simulink model "HDVSim_XNG_Hybrid_Truck"  (R2019b) (continued)
== system HDVSim_XNG_Hybrid_Truck/Driving cycle == (continued)
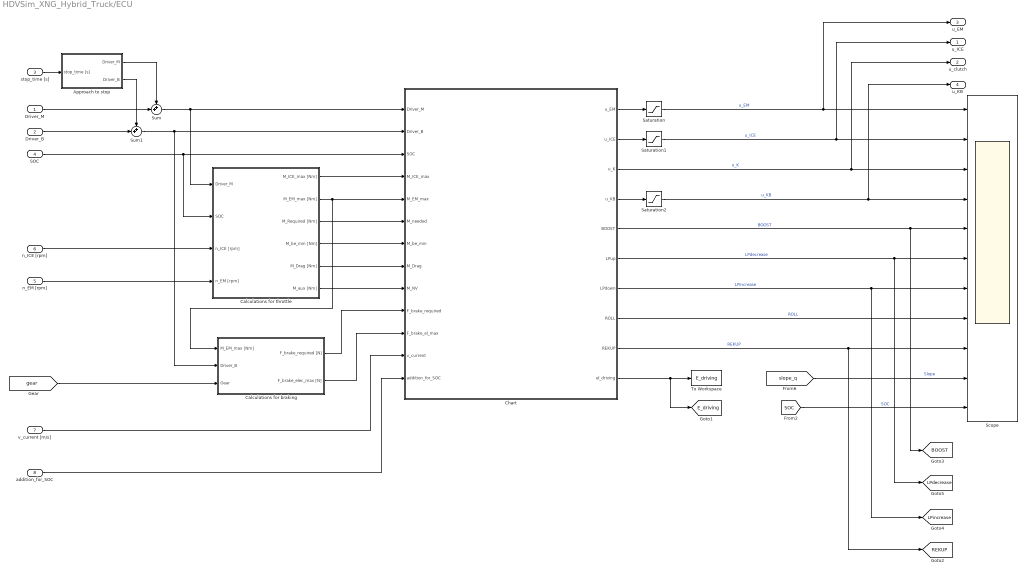
== system HDVSim_XNG_Hybrid_Truck/ECU ==
  Driver_M : Inport
  Driver_B : Inport  Port=2
  stop_time [s] : Inport  Port=3
  SOC : Inport  Port=4
  n_EM [rpm] : Inport  Port=5
  n_ICE [rpm] : Inport  Port=6
  v_current [m/s] : Inport  Port=7
  addition_for_SOC : Inport  Port=8
  Approach to stop : Subsystem
  Calculations for braking : Subsystem
  Calculations for throttle : Subsystem
  Chart : Stateflow chart  TreatAsAtomicUnit=on
  Saturation : Saturate  UpperLimit=1  LowerLimit=-1
  Saturation1 : Saturate  UpperLimit=1  LowerLimit=0
  Saturation2 : Saturate  UpperLimit=1  LowerLimit=0
  Scope : Scope  NumInputPorts=11
  Sum : Sum  Inputs=++|
  Sum1 : Sum  Inputs=++|
  To Workspace : ToWorkspace  VariableName=E_driving  SampleTime=-1
      settings: Decimation=10
  u_ICE : Outport  VectorParamsAs1DForOutWhenUnconnected=off
  u_clutch : Outport  Port=2  VectorParamsAs1DForOutWhenUnconnected=off
  u_EM : Outport  Port=3  VectorParamsAs1DForOutWhenUnconnected=off
  u_KB : Outport  Port=4  VectorParamsAs1DForOutWhenUnconnected=off
  tag scope: BOOST=global, E_driving=global, LPdecrease=global, LPincrease=global, REKUP=global
  wires:
  Chart/1(u_EM) -> Saturation/1
  Chart/2(u_ICE) -> Saturation1/1
  Chart/4(u_KB) -> Saturation2/1
  Sum1/1 -> Chart/2(Driver_B), Calculations for braking/2(Driver_B)
  Sum/1 -> Chart/1(Driver_M), Calculations for throttle/1(Driver_M)
  Chart/3(u_K) -> u_clutch/1, Scope/3  "u_K"
  Calculations for braking/2(F_brake_elec_max [N]) -> Chart/11(F_brake_el_max)
  Calculations for braking/1(F_brake_required [N]) -> Chart/10(F_brake_required)
  SOC/1 -> Chart/3(SOC), Calculations for throttle/2(SOC)
  n_ICE [rpm]/1 -> Calculations for throttle/3(n_ICE [rpm])
  Calculations for throttle/1(M_ICE_max [Nm]) -> Chart/4(M_ICE_max)
  Calculations for throttle/3(M_Required [Nm]) -> Chart/6(M_needed)
  Saturation1/1 -> u_ICE/1, Scope/2  "u_ICE"
  Saturation2/1 -> u_KB/1, Scope/4  "u_KB"
  Calculations for throttle/5(M_Drag [Nm]) -> Chart/8(M_Drag)
  Calculations for throttle/4(M_be_min [Nm]) -> Chart/7(M_be_min)
  Chart/5(BOOST) -> Scope/5, [BOOST]  "BOOST"
  Chart/8(ROLL) -> Scope/8  "ROLL"
  Chart/9(REKUP) -> [REKUP], Scope/9  "REKUP"
  Chart/7(LPdown) -> [LPincrease], Scope/7  "LPincrease"
  [slope_q] -> Scope/10  "Slope"
  Calculations for throttle/6(M_aux [Nm]) -> Chart/9(M_NV)
  Chart/6(LPup) -> [LPdecrease], Scope/6  "LPdecrease"
  Calculations for throttle/2(M_EM_max [Nm]) -> Chart/5(M_EM_max), Calculations for braking/1(M_EM_max [Nm])
  [SOC] -> Scope/11  "SOC"
  Saturation/1 -> u_EM/1, Scope/1  "u_EM"
  stop_time [s]/1 -> Approach to stop/1(stop_time [s])
  Approach to stop/2(Driver_B) -> Sum1/1
  Approach to stop/1(Driver_M) -> Sum/1
  Driver_M/1 -> Sum/2
  Driver_B/1 -> Sum1/2
  n_EM [rpm]/1 -> Calculations for throttle/4(n_EM [rpm])
  [gear] -> Calculations for braking/3(Gear)
  v_current [m/s]/1 -> Chart/12(v_current)
  Chart/10(el_driving) -> [E_driving], To Workspace/1
  addition_for_SOC/1 -> Chart/13(addition_for_SOC)

== system HDVSim_XNG_Hybrid_Truck/Electric motor ==
  note: Electric torque
  note: Effective motor power
  u_EM : Inport
  n_EM [rpm] : Inport  Port=2
  U_Bat [V] : Inport  Port=3
  Abs : Abs
  Abs1 : Abs
  Divide : Product  Inputs=*/
  Electric machine efficiency mapping : Lookup_n-D  BreakpointsForDimension1=em.efficiency.torque  BreakpointsForDimension2=em. efficiency.speed  Table=em.efficiency.characteristic_map  RndMeth=Simplest  InputSameDT=on
  Electric machine efficiency mapping2 : Lookup_n-D  BreakpointsForDimension1=em.efficiency.torque  BreakpointsForDimension2=em. efficiency.speed  Table=em.efficiency.characteristic_map  RndMeth=Simplest  InputSameDT=on
  Gain : Gain  Gain=2*pi/60
  Machine current demand : Subsystem
  Machine torque demand : Subsystem
  Math Function : Math  Operator=reciprocal  SaturateOnIntegerOverflow=on
  Product1 : Product
  Product2 : Product  Inputs=3
  Saturation : Saturate  UpperLimit=Bat.Useable_capacity / Bat.Useable_range*10  LowerLimit=-Bat.Useable_capacity / Bat.Useable_range*10
  Saturation1 : Saturate  UpperLimit=inf  LowerLimit=1
  Speed-torrque characteristics : Lookup_n-D  NumberOfTableDimensions=1  BreakpointsForDimension1=em.speed  Table=em.trq  ExtrapMethod=Clip  RndMeth=Simplest  InputSameDT=on
  Switch : Switch
  M_EM [Nm] : Outport  VectorParamsAs1DForOutWhenUnconnected=off
  I_EM_current [A] : Outport  Port=2  VectorParamsAs1DForOutWhenUnconnected=off
  tag scope: M_EM=global, n_EM=global, u_EM=global
  wires:
  Speed-torrque characteristics/1 -> Product1/1  "M_EM_max"
  u_EM/1 -> Product1/2, [u_EM]
  U_Bat [V]/1 -> Divide/2, Machine torque demand/4(U_Bat [V]), Machine current demand/4(U_Bat [V])
  Machine current demand/1(I_target [A]) -> Saturation/1
  Saturation/1 -> Machine torque demand/2(I_current [A])
  Machine torque demand/1(M_EM [Nm]) -> [M_EM], M_EM [Nm]/1, Switch/2, Abs/1, Product2/3
  Gain/1 -> Product2/2
  Divide/1 -> I_EM_current [A]/1
  Math Function/1 -> Switch/1
  Electric machine efficiency mapping2/1 -> Switch/3, Math Function/1  "Eta_EM"
  Switch/1 -> Product2/1
  Product2/1 -> Divide/1
  Abs/1 -> Electric machine efficiency mapping2/1
  Product1/1 -> Machine current demand/2(M_EM_target [Nm]), Abs1/1  "M_EM_target"
  n_EM [rpm]/1 -> Electric machine efficiency mapping/2, Saturation1/1, Electric machine efficiency mapping2/2, Machine torque demand/1(n_EM [rpm]), Machine current demand/1("n_EM "), [n_EM], Speed-torrque characteristics/1
  Abs1/1 -> Electric machine efficiency mapping/1
  Electric machine efficiency mapping/1 -> Machine current demand/3(Eff_EM), Machine torque demand/3(Eff_EM)  "ETA_EM"
  Saturation1/1 -> Gain/1

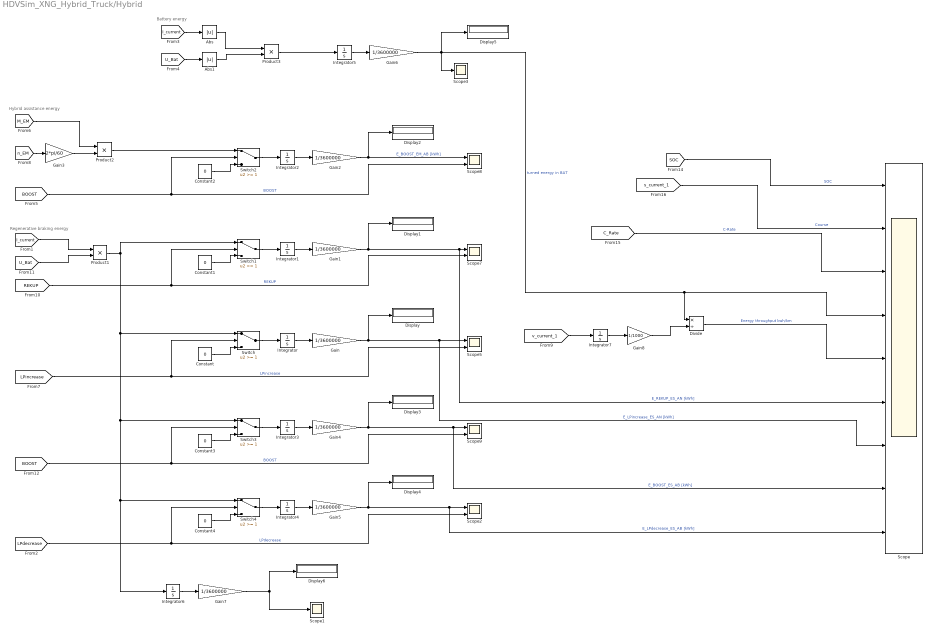
== system HDVSim_XNG_Hybrid_Truck/Hybrid ==
  note: Battery energy
  note: Hybrid assistance energy
  note: Regenerative braking energy
  Abs : Abs
  Abs1 : Abs
  Constant{,1..4} (5 alike) : Constant  Value=0
  Display{,1..6} (7 alike) : Display
  Divide : Product  Inputs=*/
  Gain{,1..2,4..7} (7 alike) : Gain  Gain=1/3600000
  Gain3 : Gain  Gain=2*pi/60
  Gain8 : Gain  Gain=1/1000
  Integrator{,1..6} (7 alike) : Integrator
  Integrator7 : Integrator  InitialCondition=0.00000001
  Product1 : Product
  Product2 : Product
  Product3 : Product
  Scope : Scope  NumInputPorts=9
  Scope1 : Scope
  Scope{2,5,7..9} (5 alike) : Scope  NumInputPorts=2
  Scope3 : Scope
  Switch{,1..4} (5 alike) : Switch  Threshold=1
  wires:
  Product1/1 -> Switch1/1, Switch/1, Switch3/1, Integrator6/1, Switch4/1
  Gain5/1 -> Scope/9, Scope2/1, Display4/1  "E_LPdecrease_ES_AB [kWh]"
  Constant4/1 -> Switch4/3
  Integrator4/1 -> Gain5/1
  Switch4/1 -> Integrator4/1
  [LPdecrease] -> Scope2/2, Switch4/2  "LPdecrease"
  Gain4/1 -> Scope/8, Scope9/1, Display3/1  "E_BOOST_ES_AB [kWh]"
  Constant3/1 -> Switch3/3
  Integrator3/1 -> Gain4/1
  Switch3/1 -> Integrator3/1
  [BOOST] -> Scope9/2, Switch3/2  "BOOST"
  Gain3/1 -> Product2/2
  [n_EM] -> Gain3/1
  Gain2/1 -> Scope8/1, Display2/1  "E_BOOST_EM_AB [kWh]"
  Constant2/1 -> Switch2/3
  Integrator2/1 -> Gain2/1
  Switch2/1 -> Integrator2/1
  [BOOST] -> Scope8/2, Switch2/2  "BOOST"
  Product2/1 -> Switch2/1
  Gain1/1 -> Scope/6, Scope7/1, Display1/1  "E_REKUP_ES_AN [kWh]"
  Constant1/1 -> Switch1/3
  Integrator1/1 -> Gain1/1
  Switch1/1 -> Integrator1/1
  [REKUP] -> Scope7/2, Switch1/2  "REKUP"
  [U_Bat] -> Product1/2
  [I_current] -> Product1/1
  Gain/1 -> Scope/7, Scope5/1, Display/1  "E_LPincrease_ES_AN [kWh]"
  Constant/1 -> Switch/3
  Integrator/1 -> Gain/1
  Switch/1 -> Integrator/1
  [LPincrease] -> Scope5/2, Switch/2  "LPincrease"
  [M_EM] -> Product2/1
  Gain7/1 -> Display6/1, Scope1/1
  Integrator6/1 -> Gain7/1
  [U_Bat] -> Abs1/1
  [I_current] -> Abs/1
  Product3/1 -> Integrator5/1
  Integrator5/1 -> Gain6/1
  Abs/1 -> Product3/1
  Abs1/1 -> Product3/2
  [SOC] -> Scope/1  "SOC"
  [s_current_1] -> Scope/2  "Course"
  [C_Rate] -> Scope/3  "C-Rate"
  Divide/1 -> Scope/5  "Energy throughput kwh/km"
  Gain6/1 -> Scope3/1, Display5/1, Divide/1, Scope/4  "turned energy in BAT"
  [v_current_1] -> Integrator7/1
  Integrator7/1 -> Gain8/1
  Gain8/1 -> Divide/2

== system HDVSim_XNG_Hybrid_Truck/ICE engine ==
  note: Engine loading
  note: Consumption calculations
  u_ICE : Inport
  n_ICE [rpm] : Inport  Port=2
  Additional consumptions : Subsystem
  Consumption : Subsystem
  Drag torque : Lookup  InputValues=engine.drag_torque.speed  Table=engine.drag_torque.trq  LookUpMeth=Interpolation-Use End Values
  Full load curve : Lookup  InputValues=engine.full_load.speed  Table=engine.full_load.trq  LookUpMeth=Interpolation-Use End Values
  MinMax : MinMax  Inputs=2
  Product1 : Product
  Sum : Sum  Inputs=|+-
  Sum1 : Sum  Inputs=|++
  maximum engine torque due to tire slip : Subsystem
  M_ICE [Nm] : Outport  VectorParamsAs1DForOutWhenUnconnected=off
  tag scope: M_mot=global, n_mot=global
  wires:
  n_ICE [rpm]/1 -> Full load curve/1, Consumption/2(n [rpm]), Additional consumptions/2(n_clutch_en [rpm]), [n_mot], Drag torque/1
  Sum/1 -> Product1/2
  Product1/1 -> Sum1/1
  Drag torque/1 -> Sum1/2, Sum/2  "M_Drag"
  Additional consumptions/1(M_ICE_aftr_aux [Nm]) -> M_ICE [Nm]/1
  u_ICE/1 -> Product1/1
  Full load curve/1 -> MinMax/2  "M_VM_max"
  Sum1/1 -> Consumption/1(M [Nm]), [M_mot], Additional consumptions/1(M_ICE_bfor_aux [Nm])
  maximum engine torque due to tire slip/1(M_mot_max_traction [Nm]) -> MinMax/1
  MinMax/1 -> Sum/1

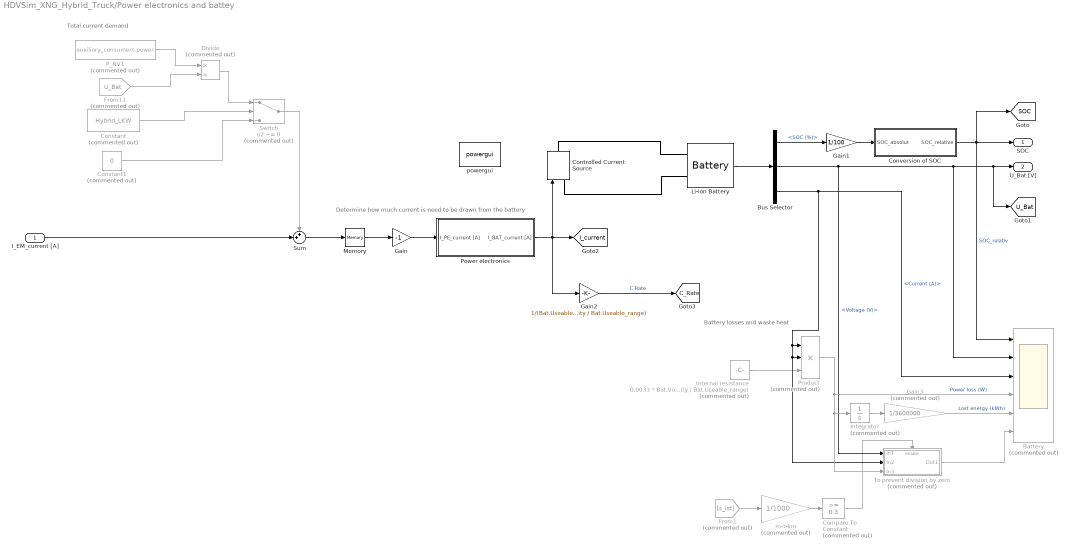
== system HDVSim_XNG_Hybrid_Truck/Power electronics and battey ==
  note: Determine how much current is need to be drawn from the battery
  note: Battery losses and waste heat
  note: Total current demand
  I_EM_current [A] : Inport
  Battery : Scope  Commented=on  NumInputPorts=6
  Bus Selector : BusSelector  OutputSignals=SOC (%),Voltage (V),Current (A)
  Compare To Constant : simulink/Logic and Bit Operations/Compare To Constant  Commented=on  relop=>=  const=0.3  OutDataTypeStr=uint8  ZeroCross=off
  Constant : Constant  Commented=on  Value=Hybrid_LKW
  Constant1 : Constant  Commented=on  Value=0
  Controlled Current Source : powerlib/Electrical Sources/Controlled Current Source  Initialize=off  Source_Type=DC  Amplitude=0  Phase=0  Frequency=0  Measurements=None
      settings: SourceProductName=Simscape Electrical
  Conversion of SOC : Subsystem
  Divide : Product  Commented=on  Inputs=*/
  From1 : From  Commented=on  GotoTag=s_ist  TagVisibility=global
      settings: CloseFcn=tagdialog Close
  From11 : From  Commented=on  GotoTag=U_Bat  TagVisibility=global
  Gain : Gain  Gain=-1
  Gain1 : Gain  Gain=1/100
  Gain2 : Gain  Gain=1/(Bat.Useable_capacity / Bat.Useable_range)
  Gain3 : Gain  Commented=on  Gain=1/3600000
  Integrator : Integrator  Commented=on
  Internal resistance : Constant  Commented=on  Value=0.0033 * Bat.Voltage / (Bat.Useable_capacity / Bat.Useable_range)
  Li-ion Battery : electricdrivelib/Extra Sources/Battery  BatType=Lithium-Ion  ShowTempParam=off  ThermalPreset=no  ShowAgeParam=off  NomV=Bat.Voltage  NomQ=Bat.Useable_capacity / Bat.Useable_range  SOC=(Bat.SOC_start *Bat.Useable_range+(0.5-Bat.Useable_range/2))* 100  Batt_Tr=30  PresetModel=off  MaxQ=Bat.Useable_capacity / Bat.Useable_range *8077/7500  MinV=487.5  FullV=Bat.Voltage * 1.178  Dis_rate=Bat.Useable_capacity / Bat.Useable_range * 1  R=0.0033 * Bat.Voltage / (Bat.Useable_capacity / Bat.Useable_range)  Normal_OP=Bat.Useable_capacity / Bat.Useable_range * 0.962  expZone="[Bat.Voltage*4339/4000     Bat.Useable_capacity / Bat.Useable_range/5]"  current=[5 25 50 250]  Units=Ampere-hour  Tci=20  NomT=20  T2=-30  MaxQ2=4.8  FullV2=7.1  Normal_OP2=5.72  expZone2="[6.58  1]"  Rca=0.6  ti=1000  Dp=0  Neq0=0  Tsim=1e6  Ta1=25  Qeol=5.4*0.9  Reol=0.013333*1.2  Char_age=[2.3478, 3]  Dis_age=[2.3478, 10]  N1=1500  N2=10500  N3=1000  N4=1400  Ta2=45  N5=950
      settings: SourceProductName=Simscape Electrical
  Memory : Memory
  P_NV1 : Constant  Commented=on  Value=auxiliary_consumers.power
  Power electronics : Subsystem
  Product : Product  Commented=on  Inputs=3
  Sum : Sum  Inputs=++|
  Switch : Switch  Commented=on  Criteria=u2 ~= 0
  To prevent division by zero : Subsystem  Commented=on
  m->km : Gain  Commented=on  Gain=1/1000
  powergui : powerlib/powergui  Priority=1  SimulationMode=Discrete  SampleTime=sample_time  frequency=60  Iterations=50  frequencyindice=0  Pbase=100e6  ErrMax=1e-4  UnitsV=kV  UnitsW=MW  buscounter=0  FunctionMessages=off  echomessages=off  EnableUseOfTLC=off  CurrentSourceSwitches=off  DisableSnubberDevices=off  DisableRonSwitches=off  DisableVfSwitches=off  DisplayEquations=off  AutomaticDiscreteSolvers=off  SolverType=Tustin  Interpol=off  ExternalGateDelay=off  methode=off  Ts=100  x0status=blocks  SPID=off  SwTol=0  HookPort=off  NonlinearTolerance=1e-4  nMaxIteration=100  ContinueOnMaxIteration=off  RestoreLinks=warning  ResistiveCurrentMeasurement=off  Frange=[0:2:500]  Ylog=off  Xlog=on  ShowGrid=off  save=off  variable=ZData  structure=0  ZoomFFT=off  StartTime=0.0  cycles=1  DisplayStyle=1  fundamental=60  FreqAxis=off  MaxFrequency=1000  frequencyindicesteady=1  RmsSteady=1  display=off
      settings: SourceProductName=Simscape Electrical
  SOC : Outport  VectorParamsAs1DForOutWhenUnconnected=off
  U_Bat [V] : Outport  Port=2  VectorParamsAs1DForOutWhenUnconnected=off
  tag scope: C_Rate=global, I_current=global, SOC=global, U_Bat=global
  wires:
  Li-ion Battery/1 -> Bus Selector/1
  Bus Selector/1 -> Gain1/1  "<SOC (%)>"
  Gain1/1 -> Conversion of SOC/1(SOC_absolut)
  Memory/1 -> Gain/1
  Gain/1 -> Power electronics/1(I_PE_current [A])
  Power electronics/1(I_BAT_current [A]) -> Gain2/1, Controlled Current Source/1, [I_current]
  Gain2/1 -> [C_Rate]  "C Rate"
  Gain3/1 -> Battery/5  "Lost energy (kWh)"
  Compare To Constant/1 -> To prevent division by zero/Enable
  To prevent division by zero/1(Out1) -> Battery/6
  Integrator/1 -> Gain3/1
  From1/1 -> m->km/1
  Internal resistance/1 -> Product/3
  Bus Selector/3 -> Battery/3, To prevent division by zero/2(In2), Product/2, Product/1  "<Current (A)>"
  Bus Selector/2 -> To prevent division by zero/1(In1), Battery/2, [U_Bat], U_Bat [V]/1  "<Voltage (V)>"
  Product/1 -> Battery/4, To prevent division by zero/3(In3), Integrator/1  "Power loss (W)"
  m->km/1 -> Compare To Constant/1
  Conversion of SOC/1(SOC_relative) -> Battery/1, [SOC], SOC/1  "SOC_relativ"
  From11/1 -> Divide/2
  P_NV1/1 -> Divide/1
  Divide/1 -> Switch/1
  Constant1/1 -> Switch/3
  Switch/1 -> Sum/1
  Constant/1 -> Switch/2
  Sum/1 -> Memory/1
  I_EM_current [A]/1 -> Sum/2
  connections:
  Li-ion Battery/LConn1 -- Controlled Current Source/RConn1
  Li-ion Battery/LConn2 -- Controlled Current Source/LConn1

== system HDVSim_XNG_Hybrid_Truck/Tires ==
  M_tire [Nm] : Inport
  v_current [m/s] : Inport  Port=2
  F=M/r : Product  Inputs=*/
  Tire radius : Constant  Value=tires.radius
  "phi. -> n[1/min]" : Gain  Gain=60/2/pi
  phi. = v/r : Product  Inputs=/*
  n_tire [rpm] : Outport  VectorParamsAs1DForOutWhenUnconnected=off
  F_x [N] : Outport  Port=2  VectorParamsAs1DForOutWhenUnconnected=off
  wires:
  phi. = v/r/1 -> "phi. -> n[1/min]"/1
  "phi. -> n[1/min]"/1 -> n_tire [rpm]/1
  Tire radius/1 -> phi. = v/r/1, F=M/r/2
  v_current [m/s]/1 -> phi. = v/r/2
  M_tire [Nm]/1 -> F=M/r/1
  F=M/r/1 -> F_x [N]/1

== system HDVSim_XNG_Hybrid_Truck/Transmission ==
  note: Transmission torque multiplication through the different gears
  M_EM [Nm] : Inport
  M_clutch_dis [Nm] : Inport  Port=2
  n_Gearbox [rpm] : Inport  Port=3
  alpha : Inport  Port=4
  Determine when shifting occurs : Subsystem  TreatAsAtomicUnit=on
  Memory1 : Memory
  Number of gears : Constant  Value=length(transmission.ratios)
  Saturation : Saturate  UpperLimit=2000  LowerLimit=0
  Shifting strategy : Stateflow chart  TreatAsAtomicUnit=on
  Sum : Sum
  Torque flow through the transmission : Subsystem
  ZKU : Subsystem
  init_gear : Constant  Value=transmission.init_gear
  n_clutch_dis [rpm] : Outport  VectorParamsAs1DForOutWhenUnconnected=off
  M_Gearbox [Nm] : Outport  Port=2  VectorParamsAs1DForOutWhenUnconnected=off
  tag scope: gear=global, i_gearbox=global, recommend_DOWN_now=global, recommend_UP_now=global
  wires:
  n_Gearbox [rpm]/1 -> Torque flow through the transmission/2(n_gearbox_out [1/min]), Determine when shifting occurs/2(n_Getr_aus [1/min])
  Torque flow through the transmission/2(M_gearbox_out [Nm]) -> [M_gear_out], M_Gearbox [Nm]/1
  Torque flow through the transmission/1(n_gearbox_in [1/min]) -> Saturation/1
  init_gear/1 -> Shifting strategy/1(init_gear)
  Determine when shifting occurs/27(check_torque_reseve_2lower) -> Shifting strategy/29(check_torque_reserve_2lower)
  Determine when shifting occurs/26(check_torque_reseve_2higher) -> Shifting strategy/28(check_torque_reserve_2higher)
  Determine when shifting occurs/1(reccommend_down_2higher) -> Shifting strategy/3(recommend_down_2higher)
  Determine when shifting occurs/2(reccommend_down_1higher) -> Shifting strategy/4(recommend_down_1higher)
  Determine when shifting occurs/3(reccommend_down_now) -> Shifting strategy/5(recommend_down_now), [recommend_DOWN_now]
  Determine when shifting occurs/4(reccommend_down_1lower) -> Shifting strategy/6(recommend_down_1lower)
  Determine when shifting occurs/5(reccommend_down_2lower) -> Shifting strategy/7(recommend_down_2llower)
  Determine when shifting occurs/6(reccommend_up_2higher) -> Shifting strategy/8(recommend_up_2higher)
  Determine when shifting occurs/7(reccommend_up_1higher) -> Shifting strategy/9(recommend_up_1higher)
  Determine when shifting occurs/8(reccommend_up_now) -> Shifting strategy/10(recommend_up_now), [recommend_UP_now]
  Determine when shifting occurs/9(reccommend_up_1lower) -> Shifting strategy/11(recommend_up_1lower)
  Determine when shifting occurs/10(reccommend_up_2lower) -> Shifting strategy/12(recommend_up_2lower)
  Determine when shifting occurs/11(check_n_min_2higher) -> Shifting strategy/13(check_n_min_2higher)
  Determine when shifting occurs/12(check_n_min_1higher) -> Shifting strategy/14(check_n_min_1higher)
  Determine when shifting occurs/13(check_n_min_now) -> Shifting strategy/15(check_n_min_now)
  Determine when shifting occurs/14(check_n_min_1lower) -> Shifting strategy/16(check_n_min_1lower)
  Determine when shifting occurs/15(check_n_min_2lower) -> Shifting strategy/17(check_n_min_2lower)
  Determine when shifting occurs/16(check_n_max_2higher) -> Shifting strategy/18(check_n_max_2higher)
  Determine when shifting occurs/17(check_n_max_1higher) -> Shifting strategy/19(check_n_max_1higher)
  Determine when shifting occurs/18(check_n_max_now) -> Shifting strategy/20(check_n_max_now)
  Determine when shifting occurs/19(check_n_max_1lower) -> Shifting strategy/21(check_n_max_1lower)
  Determine when shifting occurs/20(check_n_max_2lower) -> Shifting strategy/22(check_n_max_2lower)
  Determine when shifting occurs/21(check_M_max_2higher) -> Shifting strategy/23(check_M_max_2higher)  "check_M_max_2higher"
  Determine when shifting occurs/22(check_M_max_1higher) -> Shifting strategy/24(check_M_max_1higher)  "check_M_max_1higher"
  Determine when shifting occurs/23(check_M_max_now) -> Shifting strategy/25(check_M_max_now)  "check_M_max_now"
  Determine when shifting occurs/24(check_M_max_1lower) -> Shifting strategy/26(check_M_max_1lower)  "check_M_max_1lower"
  Determine when shifting occurs/25(check_M_max_2lower) -> Shifting strategy/27(check_M_max_2lower)  "check_M_max_2lower"
  Memory1/1 -> ZKU/1(Gear), Torque flow through the transmission/3(Gear), [Gear], [gear]
  M_clutch_dis [Nm]/1 -> Sum/2
  Sum/1 -> Torque flow through the transmission/1(M_gearbox_in [Nm])
  Torque flow through the transmission/3(i_gearbox) -> [i_gearbox]
  Saturation/1 -> n_clutch_dis [rpm]/1
  M_EM [Nm]/1 -> Determine when shifting occurs/4(M_EM [Nm]), Sum/1
  Number of gears/1 -> Shifting strategy/2(z)
  alpha/1 -> Determine when shifting occurs/5(alpha)
  Shifting strategy/1(gear) -> [gear]
  [gear] -> Memory1/1
  [Gear] -> Determine when shifting occurs/1(Gear)
  [M_gear_out] -> Determine when shifting occurs/3(M_Getr_aus [Nm])

== system HDVSim_XNG_Hybrid_Truck/Vehicle ==
  note: Net tractive force applied on the vehicle
  note: Vehicle acceleration
  F_x [N] : Inport
  u_KB : Inport  Port=2
  alpha [rad] : Inport  Port=3
  Add : Sum  Inputs=-+-
  Breaking force [N] : Product  RndMeth=Zero
  Cargo weight : Constant  Value=vehicle.payload
  Driving resistances without acceleration : Subsystem
  F_brake_max : Constant  Value=vehicle.F_brake_max
  Integrator : Integrator
  Integrator1 : Integrator  InitialCondition=cycle.speed_init  LimitOutput=on  UpperSaturationLimit=33  LowerSaturationLimit=0
  Product2 : Product  Inputs=*/  RndMeth=Zero
  Product3 : Product  Inputs=**  RndMeth=Zero
  Saturation : Saturate  UpperLimit=15  LowerLimit=-15
  Sum1 : Sum  Inputs=|++
  Vehicle weight : Constant  Value=vehicle.mass
  v_current [m/s] : Outport  VectorParamsAs1DForOutWhenUnconnected=off
  s_current [m] : Outport  Port=2  VectorParamsAs1DForOutWhenUnconnected=off
  tag scope: s_current_1=global, v_current_1=global
  wires:
  alpha [rad]/1 -> Driving resistances without acceleration/1(alpha [rad])
  Add/1 -> Product2/1
  Driving resistances without acceleration/1(F_W [N]) -> Add/3
  F_x [N]/1 -> Add/2  "F_x"
  F_brake_max/1 -> Breaking force [N]/2
  Integrator/1 -> [s_current_1], s_current [m]/1  "s_ist"
  Cargo weight/1 -> Sum1/2
  Sum1/1 -> Product2/2
  Vehicle weight/1 -> Product3/2
  Product3/1 -> Sum1/1
  Integrator1/1 -> v_current [m/s]/1, Driving resistances without acceleration/2(v [m/s]), [v_current_1], Integrator/1  "v_ist"
  [lamda] -> Product3/1
  u_KB/1 -> Breaking force [N]/1
  Breaking force [N]/1 -> Add/1  "F_brake"
  Product2/1 -> Saturation/1  "a"
  Saturation/1 -> Integrator1/1

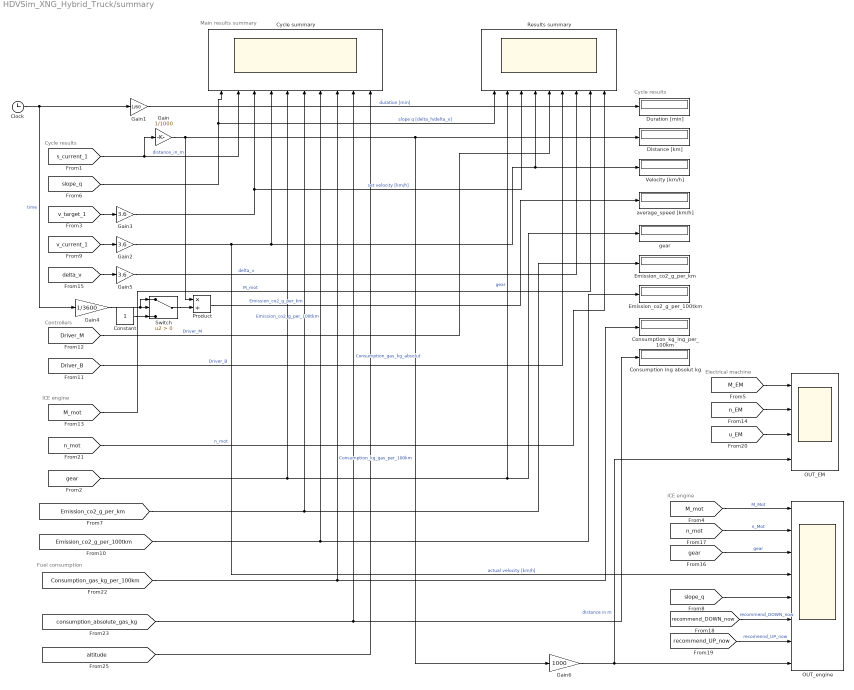
== system HDVSim_XNG_Hybrid_Truck/summary ==
  note: Main results summary
  note: Cycle results
  note: Controllers
  note: ICE engine
  note: Fuel consumption
  note: Cycle results
  note: Electrical machine
  note: ICE engine
  Clock : Clock
  Constant : Constant
  Consumption lng absolut kg : Display
  Consumption_kg_lng_per_100km : Display
  Cycle summary : Scope  NumInputPorts=10
  Distance [km] : Display
  Duration [min] : Display
  Emission_co2_g_per_100tkm : Display
  Emission_co2_g_per_km : Display
  Gain : Gain  Gain=1/1000
  Gain1 : Gain  Gain=1/60
  Gain2 : Gain  Gain=3.6
  Gain3 : Gain  Gain=3.6
  Gain4 : Gain  Gain=1/3600
  Gain5 : Gain  Gain=3.6
  Gain6 : Gain  Gain=1000
  OUT_EM : Scope  NumInputPorts=4
  OUT_engine : Scope  NumInputPorts=8
  Product : Product  Inputs=*/  RndMeth=Zero
  Results summary : Scope  NumInputPorts=9
  Switch : Switch  Criteria=u2 > Threshold
  Velocity [km/h] : Display
  average_speed [km/h] : Display
  gear : Display
  wires:
  [v_current_1] -> Gain2/1
  [s_current_1] -> Cycle summary/2, Gain/1  "distance_in_m"
  Gain/1 -> Gain6/1, Distance [km]/1, Product/1
  Clock/1 -> Gain1/1, Gain4/1  "time"
  Gain1/1 -> Duration [min]/1  "duration [min]"
  Gain2/1 -> Velocity [km/h]/1, Results summary/4, Cycle summary/4, OUT_engine/4  "actual velocity [km/h]"
  [gear] -> gear/1, Results summary/2, Cycle summary/5  "gear"
  [v_target_1] -> Gain3/1
  [slope_q] -> Results summary/1, Cycle summary/1  "slope q [delta_h/delta_x]"
  [Emission_co2_g_per_km] -> Emission_co2_g_per_km/1, Cycle summary/6  "Emission_co2_g_per_km"
  Gain3/1 -> Results summary/3, Cycle summary/3  "set velocity [km/h]"
  Gain4/1 -> Switch/1, Switch/2
  Product/1 -> average_speed [km/h]/1
  [Emission_co2_g_per_100tkm] -> Emission_co2_g_per_100tkm/1, Cycle summary/7  "Emission_co2_g_per_100tkm"
  [Driver_M] -> Results summary/5  "Driver_M"
  [Driver_B] -> Results summary/6  "Driver_B"
  [M_mot] -> Results summary/8  "M_mot"
  [delta_v] -> Gain5/1
  [M_mot] -> OUT_engine/1  "M_Mot"
  [n_mot] -> OUT_engine/2  "n_Mot"
  [gear] -> OUT_engine/3  "gear"
  [recommend_DOWN_now] -> OUT_engine/6  "recommend_DOWN_now"
  [recommend_UP_now] -> OUT_engine/7  "recomennd_UP_now"
  [slope_q] -> OUT_engine/5
  Gain5/1 -> Results summary/7  "delta_v"
  [n_mot] -> Results summary/9  "n_mot"
  [M_EM] -> OUT_EM/1
  [n_EM] -> OUT_EM/2
  [u_EM] -> OUT_EM/3
  [Consumption_gas_kg_per_100km] -> Consumption_kg_lng_per_100km/1, Cycle summary/8  "Consumption_kg_gas_per_100km"
  [consumption_absolute_gas_kg] -> Consumption lng absolut kg/1, Cycle summary/9  "Consumption_gas_kg_absolut"
  Switch/1 -> Product/2
  Constant/1 -> Switch/3
  [altitude] -> Cycle summary/10
  Gain6/1 -> OUT_EM/4, OUT_engine/8  "distance in m"

== system HDVSim_XNG_Hybrid_Truck/Active cruise control/Braking from upper speed limit ==
  v_target [m/s] : Inport
  Add3 : Sum  Inputs=+-
  Constant1 : Constant  Value=90/3.6
  Constant10 : Constant  Value=prospective_cruise_control
  Constant6 : Constant  Value=v_PPC_delta
  Constant7 : Constant  Value=0
  Constant8 : Constant  Value=0
  Saturation Dynamic : simulink/Discontinuities/Saturation Dynamic  OutMin=[]  OutMax=[]  OutDataTypeStr=Inherit: Same as second input  OutDataType=fixdt(1, 16)  OutScaling=2^-10  DoSatur=off
  Switch3 : Switch  Criteria=u2 > Threshold
  Out1 : Outport  VectorParamsAs1DForOutWhenUnconnected=off
  wires:
  Switch3/1 -> Out1/1
  Constant6/1 -> Saturation Dynamic/2
  Constant1/1 -> Add3/1
  Constant7/1 -> Switch3/3
  Add3/1 -> Saturation Dynamic/1
  Constant8/1 -> Saturation Dynamic/3
  Constant10/1 -> Switch3/2
  v_target [m/s]/1 -> Add3/2
  Saturation Dynamic/1 -> Switch3/1
